simulink model "HvacCtrlrAirFlowDistbnFrnt"  (R2022b)
settings: Solver=FixedStepDiscrete  StartTime=0.0  StopTime=10.0  FixedStep=0.2  RelTol=1e-3
(part 1 of 2)
settings Model: Creator=blue.ge  LastModifiedBy=blue.ge
settings DataIOCC: SaveFormat=StructureWithTime
settings OptimizationCC: DefaultParameterBehavior=Inlined  EnhancedBackFolding=on  PassReuseOutputArgsAs=Individual arguments  ZeroExternalMemoryAtStartup=off  ZeroInternalMemoryAtStartup=off  OptimizeBlockOrder=speed  DifferentSizesBufferReuse=on  OptimizationCustomize=off  EfficientTunableParamExpr=on
settings DebuggingCC: ParameterTunabilityLossMsg=error
settings ModelReferenceCC: ModelReferenceNumInstancesAllowed=Single
settings SFSimCC: MATLABDynamicMemAlloc=off
settings RTWCC: Description=AUTOSAR  SystemTargetFile=autosar.tlc  GenCodeOnly=on  TemplateMakefile=ert_default_tmf
settings CodeAppCC: ForceParamTrailComments=on  IgnoreCustomStorageClasses=off  OperatorAnnotations=on  SimulinkDataObjDesc=on  SFDataObjDesc=on  InsertBlockDesc=on
settings STFCustomTargetCC: TargetLangStandard=C99 (ISO)  MultiwordLength=256  ParMdlRefBuildCompliant=on  CompOptLevelCompliant=on  IncludeMdlTerminateFcn=off  CombineOutputUpdateFcns=on  SuppressErrorStatus=on  ERTCustomFileBanners=on  SupportNonFinite=off  SupportComplex=off  SupportContinuousTime=off  SupportNonInlinedSFcns=off  RemoveResetFunc=on  MATLABClassNameForMDSCustomization=Simulink.SoftwareTarget.ERTCustomization  AutosarCompliant=on  UseToolchainInfoCompliant=on  LookupTableObjectStructAxisOrder=2,1,3,4,...  LUTObjectStructOrderEvenSpacing=Size,Table,Breakpoints  SystemTargetFile=autosar.tlc
settings ERTTargetCC: TargetLangStandard=C99 (ISO)  ConcurrentExecutionCompliant=off  IncludeMdlTerminateFcn=off  SuppressErrorStatus=on  SupportNonFinite=off  SupportComplex=off  ModelStepFunctionPrototypeControlCompliant=off  CPPClassGenCompliant=off  AutosarCompliant=on  LookupTableObjectStructAxisOrder=2,1,3,4,...  LUTObjectStructOrderEvenSpacing=Size,Table,Breakpoints  InlinedParameterPlacement=NonHierarchical  ERTCustomFileTemplate=example_file_process.tlc

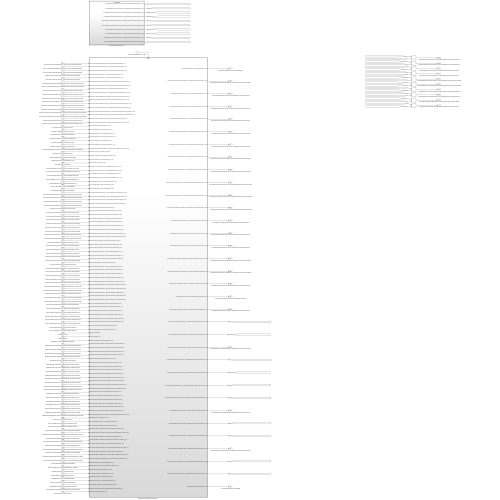
[thumbnail: Simulink's stored preview of the top-level diagram]
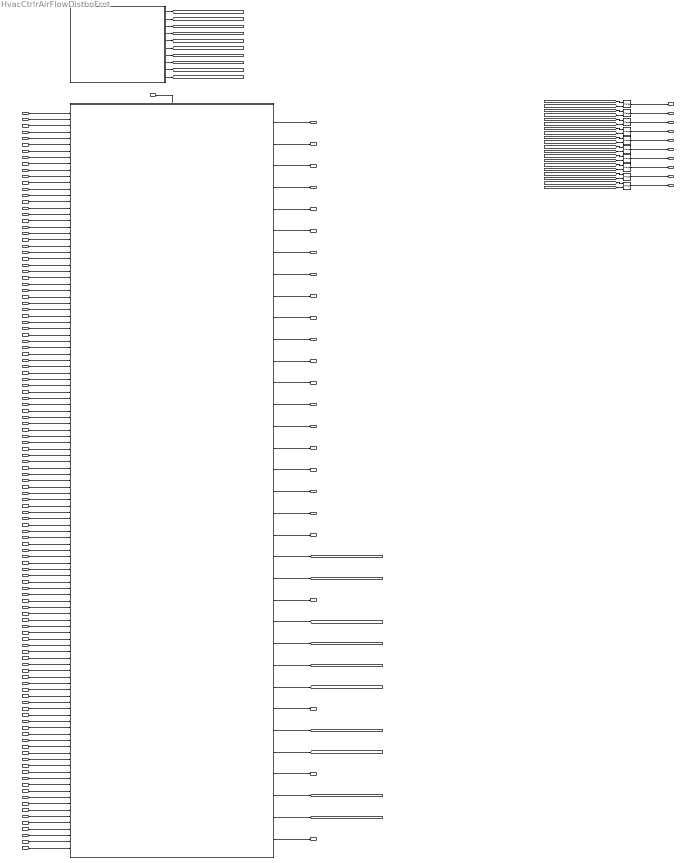
== system HvacCtrlrAirFlowDistbnFrnt ==
  HvacCtrlrAirFlowDistbnFrnt_Runnable : Inport  SampleTime=0.2  OutputFunctionCall=on
  ActrPosnFrntActForRowFirstModDistbn_ActrPosnFrntActForRowFirstModDistbn : Inport  Port=2  OutMin=0  OutMax=100  OutDataTypeStr=Perc9  PortDimensions=1
  ActrPosnFrntActForRowSecModDistbnLe_ActrPosnFrntActForRowSecModDistbnLe : Inport  Port=3  OutMin=0  OutMax=100  OutDataTypeStr=Perc9  PortDimensions=1
  ActrPosnFrntActForRowSecModDistbnRi_ActrPosnFrntActForRowSecModDistbnRi : Inport  Port=4  OutMin=0  OutMax=100  OutDataTypeStr=Perc9  PortDimensions=1
  ActrPosnMaxLimForVentForRowSec_ActrPosnMaxLimForVentForRowSec : Inport  Port=5  OutDataTypeStr=Perc30  PortDimensions=1
  ActrPosnMinLimForVentForRowSec_ActrPosnMinLimForVentForRowSec : Inport  Port=6  OutDataTypeStr=Perc30  PortDimensions=1
  ActrPosnReqForModDistbnForClimaCtrlMan_ActrPosnReqForModDistbnForClimaCtrlMan : Inport  Port=7  OutDataTypeStr=Perc30  PortDimensions=1
  AirFlapPosnFrntActForRowFirstForActrDefrst_AirFlapPosnFrntActForRowFirstForActrDefrst : Inport  Port=8  OutMin=0  OutMax=100  OutDataTypeStr=Perc9  PortDimensions=1
  AirFlapPosnFrntActForRowFirstForActrFlr_AirFlapPosnFrntActForRowFirstForActrFlr : Inport  Port=9  OutMin=0  OutMax=100  OutDataTypeStr=Perc9  PortDimensions=1
  AirFlapPosnFrntActForRowFirstForActrFlrLe_AirFlapPosnFrntActForRowFirstForActrFlrLe : Inport  Port=10  OutMin=0  OutMax=100  OutDataTypeStr=Perc9  PortDimensions=1
  AirFlapPosnFrntActForRowFirstForActrFlrRi_AirFlapPosnFrntActForRowFirstForActrFlrRi : Inport  Port=11  OutMin=0  OutMax=100  OutDataTypeStr=Perc9  PortDimensions=1
  AirFlapPosnFrntActForRowFirstForActrVent_AirFlapPosnFrntActForRowFirstForActrVent : Inport  Port=12  OutMin=0  OutMax=100  OutDataTypeStr=Perc9  PortDimensions=1
  AirFlapPosnFrntActForRowFirstForActrVentLe_AirFlapPosnFrntActForRowFirstForActrVentLe : Inport  Port=13  OutMin=0  OutMax=100  OutDataTypeStr=Perc9  PortDimensions=1
  AirFlapPosnFrntActForRowFirstForActrVentRi_AirFlapPosnFrntActForRowFirstForActrVentRi : Inport  Port=14  OutMin=0  OutMax=100  OutDataTypeStr=Perc9  PortDimensions=1
  AirFlapPosnFrntActForRowFirstForActrVentSideLe_AirFlapPosnFrntActForRowFirstForActrVentSideLe : Inport  Port=15  OutMin=0  OutMax=100  OutDataTypeStr=Perc9  PortDimensions=1
  AirFlapPosnFrntActForRowFirstForActrVentSideRi_AirFlapPosnFrntActForRowFirstForActrVentSideRi : Inport  Port=16  OutMin=0  OutMax=100  OutDataTypeStr=Perc9  PortDimensions=1
  AirFlapPosnFrntActForRowSecForActrFlr_AirFlapPosnFrntActForRowSecForActrFlr : Inport  Port=17  OutMin=0  OutMax=100  OutDataTypeStr=Perc9  PortDimensions=1
  AirFlapPosnFrntActForRowSecForActrVent_AirFlapPosnFrntActForRowSecForActrVent : Inport  Port=18  OutMin=0  OutMax=100  OutDataTypeStr=Perc9  PortDimensions=1
  AmbTFildForClimaNgcc_AmbTFildForClima : Inport  Port=19  OutDataTypeStr=T30  PortDimensions=1
  ClimaCfgForActrModVrnt1_ClimaCfgForActrInd1 : Inport  Port=20  OutDataTypeStr=Enum: ClimaCfgForActrInd1  PortDimensions=1
  ClimaCfgForActrModVrnt1_ClimaCfgForActrModVrnt1 : Inport  Port=21  OutDataTypeStr=Enum: ClimaCfgForActrModVrnt1  PortDimensions=1
  ClimaCfgForActrModVrnt1_ClimaCfgForActrModVrntCen : Inport  Port=22  OutDataTypeStr=Enum: ClimaCfgForActrModVrntCen  PortDimensions=1
  ClimaCfgForActrModVrnt2_ClimaCfgForActrInd2 : Inport  Port=23  OutDataTypeStr=Enum: ClimaCfgForActrInd2  PortDimensions=1
  ClimaCfgForActrModVrnt2_ClimaCfgForActrModVrnt2 : Inport  Port=24  OutDataTypeStr=Enum: ClimaCfgForActrModVrnt2  PortDimensions=1
  ClimaCfgForBlowrInRowSecVentSidePrsnt1_ClimaCfgForBlowrInRowSecVentSidePrsnt1 : Inport  Port=25  OutDataTypeStr=Boolean  PortDimensions=1
  ClimaCfgForCarTyp1_ClimaCfgForCarTyp1 : Inport  Port=26  OutDataTypeStr=Enum: ClimaCfgForCarTyp1  PortDimensions=1
  ClimaCfgForClimaCtrlMan1_ClimaCfgForClimaCtrlMan1 : Inport  Port=27  OutDataTypeStr=Boolean  PortDimensions=1
  ClimaCfgForNrOfTSetg1_ClimaCfgForNrOfTSetg1 : Inport  Port=28  OutDataTypeStr=Enum: ClimaCfgForNrOfTSetg1  PortDimensions=1
  ClimaCtrlActvNgcc_ClimaCtrlActv : Inport  Port=29  OutDataTypeStr=Boolean  PortDimensions=1
  HmiCmptmtAirDistbnFrntForClima_HmiCmptmtAirDistbnFrntForClima : Inport  Port=30  OutDataTypeStr=Enum: HmiCmptmtAirDistbnFrnt  PortDimensions=1
  HmiCmptmtAirDistbnFrntForClima_HmiCmptmtAirDistbnFrntRiForClima : Inport  Port=31  OutDataTypeStr=Enum: HmiCmptmtAirDistbnFrnt  PortDimensions=1
  HmiCmptmtAirDistbnFrntForClima_HmiCmptmtAirDistbnReForClima : Inport  Port=32  OutDataTypeStr=Enum: HmiCmptmtAirDistbnFrnt  PortDimensions=1
  HmiCmptmtAirDistbnFrntForClima_HmiCmptmtAirDistbnReRiForClima : Inport  Port=33  OutDataTypeStr=Enum: HmiCmptmtAirDistbnFrnt  PortDimensions=1
  HvacAirMFlowEstimdForCtrlr_HvacAirMFlowEstimdForCtrlr : Inport  Port=34  OutDataTypeStr=AirFlow30  PortDimensions=1
  HvacCtrlrFanBoostReqLe_HvacCtrlrFanBoostReqLe : Inport  Port=35  OutDataTypeStr=Perc30  PortDimensions=1
  HvacCtrlrFanBoostReqRi_HvacCtrlrFanBoostReqRi : Inport  Port=36  OutDataTypeStr=Perc30  PortDimensions=1
  HvacCtrlrReqFrntForRowFirstForActrTLe_HvacCtrlrReqFrntForRowFirstForActrTLe : Inport  Port=37  OutDataTypeStr=Perc30  PortDimensions=1
  HvacCtrlrReqFrntForRowFirstForActrTRi_HvacCtrlrReqFrntForRowFirstForActrTRi : Inport  Port=38  OutDataTypeStr=Perc30  PortDimensions=1
  HvacCtrlrReqFrntForRowSecForActrTLe_HvacCtrlrReqFrntForRowSecForActrTLe : Inport  Port=39  OutDataTypeStr=Perc30  PortDimensions=1
  HvacCtrlrReqFrntForRowSecForActrTRi_HvacCtrlrReqFrntForRowSecForActrTRi : Inport  Port=40  OutDataTypeStr=Perc30  PortDimensions=1
  HvacFlowFrntActForDefrst_HvacFlowFrntActForDefrst : Inport  Port=41  OutDataTypeStr=AirFlow30  PortDimensions=1
  HvacFlowFrntActForRowFirstAtFlr_HvacFlowFrntActForRowFirstAtFlr : Inport  Port=42  OutDataTypeStr=AirFlow30  PortDimensions=1
  HvacFlowFrntActForRowFirstAtFlr_HvacFlowFrntActForRowFirstAtFlrLe : Inport  Port=43  OutDataTypeStr=AirFlow30  PortDimensions=1
  HvacFlowFrntActForRowFirstAtFlr_HvacFlowFrntActForRowFirstAtFlrRi : Inport  Port=44  OutDataTypeStr=AirFlow30  PortDimensions=1
  HvacFlowFrntActForRowFirstAtVent_HvacFlowFrntActForRowFirstAtVent : Inport  Port=45  OutDataTypeStr=AirFlow30  PortDimensions=1
  HvacFlowFrntActForRowFirstAtVent_HvacFlowFrntActForRowFirstAtVentLe : Inport  Port=46  OutDataTypeStr=AirFlow30  PortDimensions=1
  HvacFlowFrntActForRowFirstAtVent_HvacFlowFrntActForRowFirstAtVentRi : Inport  Port=47  OutDataTypeStr=AirFlow30  PortDimensions=1
  HvacFlowFrntActForRowFirstAtVent_HvacFlowFrntActForRowFirstAtVentSideLe : Inport  Port=48  OutDataTypeStr=AirFlow30  PortDimensions=1
  HvacFlowFrntActForRowFirstAtVent_HvacFlowFrntActForRowFirstAtVentSideRi : Inport  Port=49  OutDataTypeStr=AirFlow30  PortDimensions=1
  HvacFlowFrntActForRowSecAtFlr_HvacFlowFrntActForRowSecAtFlr : Inport  Port=50  OutDataTypeStr=AirFlow30  PortDimensions=1
  HvacFlowFrntActForRowSecAtFlr_HvacFlowFrntActForRowSecAtFlrLe : Inport  Port=51  OutDataTypeStr=AirFlow30  PortDimensions=1
  HvacFlowFrntActForRowSecAtFlr_HvacFlowFrntActForRowSecAtFlrRi : Inport  Port=52  OutDataTypeStr=AirFlow30  PortDimensions=1
  HvacFlowFrntActForRowSecAtVent_HvacFlowFrntActForRowSecAtVent : Inport  Port=53  OutDataTypeStr=AirFlow30  PortDimensions=1
  HvacFlowFrntActForRowSecAtVent_HvacFlowFrntActForRowSecAtVentLe : Inport  Port=54  OutDataTypeStr=AirFlow30  PortDimensions=1
  HvacFlowFrntActForRowSecAtVent_HvacFlowFrntActForRowSecAtVentRi : Inport  Port=55  OutDataTypeStr=AirFlow30  PortDimensions=1
  HvacFlowFrntReqForDefrst_HvacFlowFrntReqForDefrst : Inport  Port=56  OutDataTypeStr=AirFlow30  PortDimensions=1
  HvacFlowFrntReqForRowFirstAtFlr_HvacFlowFrntReqForRowFirstAtFlr : Inport  Port=57  OutDataTypeStr=AirFlow30  PortDimensions=1
  HvacFlowFrntReqForRowFirstAtFlr_HvacFlowFrntReqForRowFirstAtFlrLe : Inport  Port=58  OutDataTypeStr=AirFlow30  PortDimensions=1
  HvacFlowFrntReqForRowFirstAtFlr_HvacFlowFrntReqForRowFirstAtFlrRi : Inport  Port=59  OutDataTypeStr=AirFlow30  PortDimensions=1
  HvacFlowFrntReqForRowFirstAtVent_HvacFlowFrntReqForRowFirstAtVent : Inport  Port=60  OutDataTypeStr=AirFlow30  PortDimensions=1
  HvacFlowFrntReqForRowFirstAtVent_HvacFlowFrntReqForRowFirstAtVentLe : Inport  Port=61  OutDataTypeStr=AirFlow30  PortDimensions=1
  HvacFlowFrntReqForRowFirstAtVent_HvacFlowFrntReqForRowFirstAtVentLeMid : Inport  Port=62  OutDataTypeStr=AirFlow30  PortDimensions=1
  HvacFlowFrntReqForRowFirstAtVent_HvacFlowFrntReqForRowFirstAtVentLeSide : Inport  Port=63  OutDataTypeStr=AirFlow30  PortDimensions=1
  HvacFlowFrntReqForRowFirstAtVent_HvacFlowFrntReqForRowFirstAtVentRi : Inport  Port=64  OutDataTypeStr=AirFlow30  PortDimensions=1
  HvacFlowFrntReqForRowFirstAtVent_HvacFlowFrntReqForRowFirstAtVentRiMid : Inport  Port=65  OutDataTypeStr=AirFlow30  PortDimensions=1
  HvacFlowFrntReqForRowFirstAtVent_HvacFlowFrntReqForRowFirstAtVentRiSide : Inport  Port=66  OutDataTypeStr=AirFlow30  PortDimensions=1
  HvacFlowFrntReqForRowSecAtFlr_HvacFlowFrntReqForRowSecAtFlr : Inport  Port=67  OutDataTypeStr=AirFlow30  PortDimensions=1
  HvacFlowFrntReqForRowSecAtFlr_HvacFlowFrntReqForRowSecAtFlrLe : Inport  Port=68  OutDataTypeStr=AirFlow30  PortDimensions=1
  HvacFlowFrntReqForRowSecAtFlr_HvacFlowFrntReqForRowSecAtFlrRi : Inport  Port=69  OutDataTypeStr=AirFlow30  PortDimensions=1
  HvacFlowFrntReqForRowSecAtVent_HvacFlowFrntReqForRowSecAtVent : Inport  Port=70  OutDataTypeStr=AirFlow30  PortDimensions=1
  HvacFlowFrntReqForRowSecAtVent_HvacFlowFrntReqForRowSecAtVentLe : Inport  Port=71  OutDataTypeStr=AirFlow30  PortDimensions=1
  HvacFlowFrntReqForRowSecAtVent_HvacFlowFrntReqForRowSecAtVentRi : Inport  Port=72  OutDataTypeStr=AirFlow30  PortDimensions=1
  HvacFlrLeakReqForRowFirst_HvacFlrLeakReqForRowFirst : Inport  Port=73  OutDataTypeStr=Boolean  PortDimensions=1
  HvacFlrLeakReqForRowSec_HvacFlrLeakReqForRowSec : Inport  Port=74  OutDataTypeStr=Boolean  PortDimensions=1
  LdAdVal_CurrAdVal : Inport  Port=75  OutDataTypeStr=Bus: LdAd  PortDimensions=1  BusOutputAsStruct=on
  LdAdVal_ValidAdVal : Inport  Port=76  OutDataTypeStr=Bus: LdAd  PortDimensions=1  BusOutputAsStruct=on
  ManModDurgFanOffActv_ManModDurgFanOffActv : Inport  Port=77  OutDataTypeStr=Boolean  PortDimensions=1
  ManModeForRowFirstLeForModelDial_ManModeForRowFirstLeForModelDial : Inport  Port=78  OutDataTypeStr=Enum: HmiCmptmtAirDistbnFrnt  PortDimensions=1
  ManModeForRowFirstRiForModelDial_ManModeForRowFirstRiForModelDial : Inport  Port=79  OutDataTypeStr=Enum: HmiCmptmtAirDistbnFrnt  PortDimensions=1
  ManModeForRowSecLeForModelDial_ManModeForRowSecLeForModelDial : Inport  Port=80  OutDataTypeStr=Enum: HmiCmptmtAirDistbnFrnt  PortDimensions=1
  ManModeForRowSecRiForModelDial_ManModeForRowSecRiForModelDial : Inport  Port=81  OutDataTypeStr=Enum: HmiCmptmtAirDistbnFrnt  PortDimensions=1
  MaxReqReqdFrntForDefrst_MaxReqReqdFrntForDefrst : Inport  Port=82  OutDataTypeStr=Boolean  PortDimensions=1
  MaxReqReqdFrntForRowFirstAtFlr_MaxReqReqdFrntForRowFirstAtFlr : Inport  Port=83  OutDataTypeStr=Boolean  PortDimensions=1
  MaxReqReqdFrntForRowFirstAtFlr_MaxReqReqdFrntForRowFirstAtFlrLe : Inport  Port=84  OutDataTypeStr=Boolean  PortDimensions=1
  MaxReqReqdFrntForRowFirstAtFlr_MaxReqReqdFrntForRowFirstAtFlrRi : Inport  Port=85  OutDataTypeStr=Boolean  PortDimensions=1
  MaxReqReqdFrntForRowFirstAtVent_MaxReqReqdFrntForRowFirstAtVent : Inport  Port=86  OutDataTypeStr=Boolean  PortDimensions=1
  MaxReqReqdFrntForRowFirstAtVent_MaxReqReqdFrntForRowFirstAtVentLe : Inport  Port=87  OutDataTypeStr=Boolean  PortDimensions=1
  MaxReqReqdFrntForRowFirstAtVent_MaxReqReqdFrntForRowFirstAtVentRi : Inport  Port=88  OutDataTypeStr=Boolean  PortDimensions=1
  MaxReqReqdFrntForRowFirstAtVent_MaxReqReqdFrntForRowFirstAtVentSideLe : Inport  Port=89  OutDataTypeStr=Boolean  PortDimensions=1
  MaxReqReqdFrntForRowFirstAtVent_MaxReqReqdFrntForRowFirstAtVentSideRi : Inport  Port=90  OutDataTypeStr=Boolean  PortDimensions=1
  MaxReqReqdFrntForRowSecAtFlr_MaxReqReqdFrntForRowSecAtFlr : Inport  Port=91  OutDataTypeStr=Boolean  PortDimensions=1
  MaxReqReqdFrntForRowSecAtFlr_MaxReqReqdFrntForRowSecAtFlrLe : Inport  Port=92  OutDataTypeStr=Boolean  PortDimensions=1
  MaxReqReqdFrntForRowSecAtFlr_MaxReqReqdFrntForRowSecAtFlrRi : Inport  Port=93  OutDataTypeStr=Boolean  PortDimensions=1
  MaxReqReqdFrntForRowSecAtVent_MaxReqReqdFrntForRowSecAtVent : Inport  Port=94  OutDataTypeStr=Boolean  PortDimensions=1
  MaxReqReqdFrntForRowSecAtVent_MaxReqReqdFrntForRowSecAtVentLe : Inport  Port=95  OutDataTypeStr=Boolean  PortDimensions=1
  MaxReqReqdFrntForRowSecAtVent_MaxReqReqdFrntForRowSecAtVentRi : Inport  Port=96  OutDataTypeStr=Boolean  PortDimensions=1
  NotAllwdForMaxReqReqdForRowSecAtVent_NotAllwdForMaxReqReqdForRowSecAtVent : Inport  Port=97  OutDataTypeStr=Boolean  PortDimensions=1
  VehSpdLgtForClima_VehSpdLgtForClima : Inport  Port=98  OutDataTypeStr=Bus: VehSpdLgtForClima  PortDimensions=1  BusOutputAsStruct=on
  VehSunLoadAtRowFirstLeFild_VehSunLoadAtRowFirstLeFild : Inport  Port=99  OutDataTypeStr=SunLoad30  PortDimensions=1
  VehSunLoadAtRowFirstRiFild_VehSunLoadAtRowFirstRiFild : Inport  Port=100  OutDataTypeStr=SunLoad30  PortDimensions=1
  ActrFrntReqForRowFirstLeModDistbn_ActrFrntReqForRowFirstLeModDistbn : Inport  Port=101  OutMin=0  OutMax=100  OutDataTypeStr=Perc9  PortDimensions=1
  ActrFrntReqForRowFirstLeModDistbn_ActrFrntReqForRowFirstLeModDistbn_IsUpdated : Inport  Port=102  OutDataTypeStr=boolean  PortDimensions=1
  ActrFrntReqForRowFirstModDistbn_ActrFrntReqForRowFirstModDistbn : Inport  Port=103  OutMin=0  OutMax=100  OutDataTypeStr=Perc9  PortDimensions=1
  ActrFrntReqForRowFirstModDistbn_ActrFrntReqForRowFirstModDistbn_IsUpdated : Inport  Port=104  OutDataTypeStr=boolean  PortDimensions=1
  ActrFrntReqForRowSecModDistbnLe_ActrFrntReqForRowSecModDistbnLe : Inport  Port=105  OutMin=0  OutMax=100  OutDataTypeStr=Perc9  PortDimensions=1
  ActrFrntReqForRowSecModDistbnLe_ActrFrntReqForRowSecModDistbnLe_IsUpdated : Inport  Port=106  OutDataTypeStr=boolean  PortDimensions=1
  ActrFrntReqForRowSecModDistbnRi_ActrFrntReqForRowSecModDistbnRi : Inport  Port=107  OutMin=0  OutMax=100  OutDataTypeStr=Perc9  PortDimensions=1
  ActrFrntReqForRowSecModDistbnRi_ActrFrntReqForRowSecModDistbnRi_IsUpdated : Inport  Port=108  OutDataTypeStr=boolean  PortDimensions=1
  ActrPosnFrntActForRowFirstModDistbnLe_ActrPosnFrntActForRowFirstModDistbnLe : Inport  Port=109  OutMin=0  OutMax=100  OutDataTypeStr=Perc9  PortDimensions=1
  ActrReqForModDistbnRe_ActrReqForModDistbnRe : Inport  Port=110  OutMin=0  OutMax=100  OutDataTypeStr=Perc9  PortDimensions=1
  ActrReqForModDistbnRe_ActrReqForModDistbnRe_IsUpdated : Inport  Port=111  OutDataTypeStr=boolean  PortDimensions=1
  ClimaAtRowSecActvNgcc_ClimaAtRowSecActv : Inport  Port=112  OutDataTypeStr=Boolean  PortDimensions=1
  ClimaAtRowSecActvNgcc_ClimaAtRowSecActvLe : Inport  Port=113  OutDataTypeStr=Boolean  PortDimensions=1
  ClimaAtRowSecActvNgcc_ClimaAtRowSecActvRi : Inport  Port=114  OutDataTypeStr=Boolean  PortDimensions=1
  ClimaCfgForHvacRePrsnt1_ClimaCfgForHvacRePrsnt1 : Inport  Port=115  OutDataTypeStr=Enum: CarCfgForHvacRe  PortDimensions=1
  ClimaCfgReqVersionReplace_ClimaCfgReqVersionReplace : Inport  Port=116  OutDataTypeStr=UInt8  PortDimensions=1
  ManModeForRowThrdForModelDial_ManModeForRowThrdForModelDial : Inport  Port=117  OutDataTypeStr=Enum: HmiCmptmtAirDistbnFrnt  PortDimensions=1
  PrkgClimaOnReq_PrkgClimaOnReq : Inport  Port=118  OutDataTypeStr=Boolean  PortDimensions=1
  HvacCtrlrAirFlowDistbnFrnt_Init : Subsystem
  HvacCtrlrAirFlowDistbnFrnt_Runnable_sys : Subsystem
  OvrdForMaxReqForActrForRowFirstAtFlr_OvrdForMaxReqForActrForRowFirstAtFlrLe_merge : Merge
  OvrdForMaxReqForActrForRowFirstAtFlr_OvrdForMaxReqForActrForRowFirstAtFlrRi_merge : Merge
  OvrdForMaxReqForActrForRowFirstAtVent_OvrdForMaxReqForActrForRowFirstAtVentLe_merge : Merge
  OvrdForMaxReqForActrForRowFirstAtVent_OvrdForMaxReqForActrForRowFirstAtVentRi_merge : Merge
  OvrdForMaxReqForActrForRowFirstAtVent_OvrdForMaxReqForActrForRowFirstAtVentSideLe_merge : Merge
  OvrdForMaxReqForActrForRowFirstAtVent_OvrdForMaxReqForActrForRowFirstAtVentSideRi_merge : Merge
  OvrdForMaxReqForActrForRowSecAtFlr_OvrdForMaxReqForActrForRowSecAtFlrLe_merge : Merge
  OvrdForMaxReqForActrForRowSecAtFlr_OvrdForMaxReqForActrForRowSecAtFlrRi_merge : Merge
  OvrdForMaxReqForActrForRowSecAtVent_OvrdForMaxReqForActrForRowSecAtVentLe_merge : Merge
  OvrdForMaxReqForActrForRowSecAtVent_OvrdForMaxReqForActrForRowSecAtVentRi_merge : Merge
  OvrdForMaxReqForActrForRowFirstAtFlr_OvrdForMaxReqForActrForRowFirstAtFlrLe : Outport  OutDataTypeStr=Boolean  PortDimensions=1
  OvrdForMaxReqForActrForRowFirstAtFlr_OvrdForMaxReqForActrForRowFirstAtFlrRi : Outport  Port=2  OutDataTypeStr=Boolean  PortDimensions=1
  OvrdForMaxReqForActrForRowFirstAtVent_OvrdForMaxReqForActrForRowFirstAtVentLe : Outport  Port=3  OutDataTypeStr=Boolean  PortDimensions=1
  OvrdForMaxReqForActrForRowFirstAtVent_OvrdForMaxReqForActrForRowFirstAtVentRi : Outport  Port=4  OutDataTypeStr=Boolean  PortDimensions=1
  OvrdForMaxReqForActrForRowFirstAtVent_OvrdForMaxReqForActrForRowFirstAtVentSideLe : Outport  Port=5  OutDataTypeStr=Boolean  PortDimensions=1
  OvrdForMaxReqForActrForRowFirstAtVent_OvrdForMaxReqForActrForRowFirstAtVentSideRi : Outport  Port=6  OutDataTypeStr=Boolean  PortDimensions=1
  OvrdForMaxReqForActrForRowSecAtFlr_OvrdForMaxReqForActrForRowSecAtFlrLe : Outport  Port=7  OutDataTypeStr=Boolean  PortDimensions=1
  OvrdForMaxReqForActrForRowSecAtFlr_OvrdForMaxReqForActrForRowSecAtFlrRi : Outport  Port=8  OutDataTypeStr=Boolean  PortDimensions=1
  OvrdForMaxReqForActrForRowSecAtVent_OvrdForMaxReqForActrForRowSecAtVentLe : Outport  Port=9  OutDataTypeStr=Boolean  PortDimensions=1
  OvrdForMaxReqForActrForRowSecAtVent_OvrdForMaxReqForActrForRowSecAtVentRi : Outport  Port=10  OutDataTypeStr=Boolean  PortDimensions=1
  HvacAirMFlowReqMaxAvl_HvacAirMFlowReqMaxAvl : Outport  Port=11  OutDataTypeStr=AirFlow30  PortDimensions=1
  HvacCtrlrReqFrntForRowFirstForActrDefrst_HvacCtrlrReqFrntForRowFirstForActrDefrst : Outport  Port=12  OutDataTypeStr=Perc30  PortDimensions=1
  HvacCtrlrReqFrntForRowFirstForActrFlr_HvacCtrlrReqFrntForRowFirstForActrFlr : Outport  Port=13  OutDataTypeStr=Perc30  PortDimensions=1
  HvacCtrlrReqFrntForRowFirstForActrFlr_HvacCtrlrReqFrntForRowFirstForActrFlrLe : Outport  Port=14  OutDataTypeStr=Perc30  PortDimensions=1
  HvacCtrlrReqFrntForRowFirstForActrFlr_HvacCtrlrReqFrntForRowFirstForActrFlrRi : Outport  Port=15  OutDataTypeStr=Perc30  PortDimensions=1
  HvacCtrlrReqFrntForRowFirstForActrMod_HvacCtrlrReqFrntForRowFirstForActrMod : Outport  Port=16  OutDataTypeStr=Perc30  PortDimensions=1
  HvacCtrlrReqFrntForRowFirstForActrVent_HvacCtrlrReqFrntForRowFirstForActrVent : Outport  Port=17  OutDataTypeStr=Perc30  PortDimensions=1
  HvacCtrlrReqFrntForRowFirstForActrVent_HvacCtrlrReqFrntForRowFirstForActrVentLe : Outport  Port=18  OutDataTypeStr=Perc30  PortDimensions=1
  HvacCtrlrReqFrntForRowFirstForActrVent_HvacCtrlrReqFrntForRowFirstForActrVentRi : Outport  Port=19  OutDataTypeStr=Perc30  PortDimensions=1
  HvacCtrlrReqFrntForRowFirstForActrVent_HvacCtrlrReqFrntForRowFirstForActrVentSideLe : Outport  Port=20  OutDataTypeStr=Perc30  PortDimensions=1
  HvacCtrlrReqFrntForRowFirstForActrVent_HvacCtrlrReqFrntForRowFirstForActrVentSideRi : Outport  Port=21  OutDataTypeStr=Perc30  PortDimensions=1
  HvacCtrlrReqFrntForRowFirstLeForActrMod_HvacCtrlrReqFrntForRowFirstLeForActrMod : Outport  Port=22  OutDataTypeStr=Perc30  PortDimensions=1
  HvacCtrlrReqFrntForRowSecForActrFlr_HvacCtrlrReqFrntForRowSecForActrFlr : Outport  Port=23  OutDataTypeStr=Perc30  PortDimensions=1
  HvacCtrlrReqFrntForRowSecForActrFlr_HvacCtrlrReqFrntForRowSecForActrFlrLe : Outport  Port=24  OutDataTypeStr=Perc30  PortDimensions=1
  HvacCtrlrReqFrntForRowSecForActrFlr_HvacCtrlrReqFrntForRowSecForActrFlrRi : Outport  Port=25  OutDataTypeStr=Perc30  PortDimensions=1
  HvacCtrlrReqFrntForRowSecForActrModLe_HvacCtrlrReqFrntForRowSecForActrModLe : Outport  Port=26  OutDataTypeStr=Perc30  PortDimensions=1
  HvacCtrlrReqFrntForRowSecForActrModRi_HvacCtrlrReqFrntForRowSecForActrModRi : Outport  Port=27  OutDataTypeStr=Perc30  PortDimensions=1
  HvacCtrlrReqFrntForRowSecForActrVent_HvacCtrlrReqFrntForRowSecForActrVent : Outport  Port=28  OutDataTypeStr=Perc30  PortDimensions=1
  OvrdForMaxReqForActrForDefrst_OvrdForMaxReqForActrForDefrst : Outport  Port=29  OutDataTypeStr=Boolean  PortDimensions=1
  OvrdForMaxReqForActrForRowFirstAtFlr_OvrdForMaxReqForActrForRowFirstAtFlr : Outport  Port=30  OutDataTypeStr=Boolean  PortDimensions=1
  OvrdForMaxReqForActrForRowFirstAtVent_OvrdForMaxReqForActrForRowFirstAtVent : Outport  Port=31  OutDataTypeStr=Boolean  PortDimensions=1
  OvrdForMaxReqForActrForRowSecAtFlr_OvrdForMaxReqForActrForRowSecAtFlr : Outport  Port=32  OutDataTypeStr=Boolean  PortDimensions=1
  OvrdForMaxReqForActrForRowSecAtVent_OvrdForMaxReqForActrForRowSecAtVent : Outport  Port=33  OutDataTypeStr=Boolean  PortDimensions=1
  ResrvdSigForECM4_ResrvdSigForECM4 : Outport  Port=34  OutDataTypeStr=BattSnsrChksTbl  PortDimensions=1
  wires:
  ActrPosnFrntActForRowFirstModDistbn_ActrPosnFrntActForRowFirstModDistbn/1 -> HvacCtrlrAirFlowDistbnFrnt_Runnable_sys/1(ActrPosnFrntActForRowFirstModDistbn_ActrPosnFrntActForRowFirstModDistbn_read)
  ActrPosnFrntActForRowSecModDistbnLe_ActrPosnFrntActForRowSecModDistbnLe/1 -> HvacCtrlrAirFlowDistbnFrnt_Runnable_sys/2(ActrPosnFrntActForRowSecModDistbnLe_ActrPosnFrntActForRowSecModDistbnLe_read)
  ActrPosnFrntActForRowSecModDistbnRi_ActrPosnFrntActForRowSecModDistbnRi/1 -> HvacCtrlrAirFlowDistbnFrnt_Runnable_sys/3(ActrPosnFrntActForRowSecModDistbnRi_ActrPosnFrntActForRowSecModDistbnRi_read)
  ActrPosnMaxLimForVentForRowSec_ActrPosnMaxLimForVentForRowSec/1 -> HvacCtrlrAirFlowDistbnFrnt_Runnable_sys/4(ActrPosnMaxLimForVentForRowSec_ActrPosnMaxLimForVentForRowSec_read)
  ActrPosnMinLimForVentForRowSec_ActrPosnMinLimForVentForRowSec/1 -> HvacCtrlrAirFlowDistbnFrnt_Runnable_sys/5(ActrPosnMinLimForVentForRowSec_ActrPosnMinLimForVentForRowSec_read)
  ActrPosnReqForModDistbnForClimaCtrlMan_ActrPosnReqForModDistbnForClimaCtrlMan/1 -> HvacCtrlrAirFlowDistbnFrnt_Runnable_sys/6(ActrPosnReqForModDistbnForClimaCtrlMan_ActrPosnReqForModDistbnForClimaCtrlMan_read)
  AirFlapPosnFrntActForRowFirstForActrDefrst_AirFlapPosnFrntActForRowFirstForActrDefrst/1 -> HvacCtrlrAirFlowDistbnFrnt_Runnable_sys/7(AirFlapPosnFrntActForRowFirstForActrDefrst_AirFlapPosnFrntActForRowFirstForActrDefrst_read)
  AirFlapPosnFrntActForRowFirstForActrFlr_AirFlapPosnFrntActForRowFirstForActrFlr/1 -> HvacCtrlrAirFlowDistbnFrnt_Runnable_sys/8(AirFlapPosnFrntActForRowFirstForActrFlr_AirFlapPosnFrntActForRowFirstForActrFlr_read)
  AirFlapPosnFrntActForRowFirstForActrFlrLe_AirFlapPosnFrntActForRowFirstForActrFlrLe/1 -> HvacCtrlrAirFlowDistbnFrnt_Runnable_sys/9(AirFlapPosnFrntActForRowFirstForActrFlrLe_AirFlapPosnFrntActForRowFirstForActrFlrLe_read)
  AirFlapPosnFrntActForRowFirstForActrFlrRi_AirFlapPosnFrntActForRowFirstForActrFlrRi/1 -> HvacCtrlrAirFlowDistbnFrnt_Runnable_sys/10(AirFlapPosnFrntActForRowFirstForActrFlrRi_AirFlapPosnFrntActForRowFirstForActrFlrRi_read)
  AirFlapPosnFrntActForRowFirstForActrVent_AirFlapPosnFrntActForRowFirstForActrVent/1 -> HvacCtrlrAirFlowDistbnFrnt_Runnable_sys/11(AirFlapPosnFrntActForRowFirstForActrVent_AirFlapPosnFrntActForRowFirstForActrVent_read)
  AirFlapPosnFrntActForRowFirstForActrVentLe_AirFlapPosnFrntActForRowFirstForActrVentLe/1 -> HvacCtrlrAirFlowDistbnFrnt_Runnable_sys/12(AirFlapPosnFrntActForRowFirstForActrVentLe_AirFlapPosnFrntActForRowFirstForActrVentLe_read)
  AirFlapPosnFrntActForRowFirstForActrVentRi_AirFlapPosnFrntActForRowFirstForActrVentRi/1 -> HvacCtrlrAirFlowDistbnFrnt_Runnable_sys/13(AirFlapPosnFrntActForRowFirstForActrVentRi_AirFlapPosnFrntActForRowFirstForActrVentRi_read)
  AirFlapPosnFrntActForRowFirstForActrVentSideLe_AirFlapPosnFrntActForRowFirstForActrVentSideLe/1 -> HvacCtrlrAirFlowDistbnFrnt_Runnable_sys/14(AirFlapPosnFrntActForRowFirstForActrVentSideLe_AirFlapPosnFrntActForRowFirstForActrVentSideLe_read)
  AirFlapPosnFrntActForRowFirstForActrVentSideRi_AirFlapPosnFrntActForRowFirstForActrVentSideRi/1 -> HvacCtrlrAirFlowDistbnFrnt_Runnable_sys/15(AirFlapPosnFrntActForRowFirstForActrVentSideRi_AirFlapPosnFrntActForRowFirstForActrVentSideRi_read)
  AirFlapPosnFrntActForRowSecForActrFlr_AirFlapPosnFrntActForRowSecForActrFlr/1 -> HvacCtrlrAirFlowDistbnFrnt_Runnable_sys/16(AirFlapPosnFrntActForRowSecForActrFlr_AirFlapPosnFrntActForRowSecForActrFlr_read)
  AirFlapPosnFrntActForRowSecForActrVent_AirFlapPosnFrntActForRowSecForActrVent/1 -> HvacCtrlrAirFlowDistbnFrnt_Runnable_sys/17(AirFlapPosnFrntActForRowSecForActrVent_AirFlapPosnFrntActForRowSecForActrVent_read)
  AmbTFildForClimaNgcc_AmbTFildForClima/1 -> HvacCtrlrAirFlowDistbnFrnt_Runnable_sys/18(AmbTFildForClimaNgcc_AmbTFildForClima_read)
  ClimaCfgForActrModVrnt1_ClimaCfgForActrInd1/1 -> HvacCtrlrAirFlowDistbnFrnt_Runnable_sys/19(ClimaCfgForActrModVrnt1_ClimaCfgForActrInd1_read)
  ClimaCfgForActrModVrnt1_ClimaCfgForActrModVrnt1/1 -> HvacCtrlrAirFlowDistbnFrnt_Runnable_sys/20(ClimaCfgForActrModVrnt1_ClimaCfgForActrModVrnt1_read)
  ClimaCfgForActrModVrnt1_ClimaCfgForActrModVrntCen/1 -> HvacCtrlrAirFlowDistbnFrnt_Runnable_sys/21(ClimaCfgForActrModVrnt1_ClimaCfgForActrModVrntCen_read)
  ClimaCfgForActrModVrnt2_ClimaCfgForActrInd2/1 -> HvacCtrlrAirFlowDistbnFrnt_Runnable_sys/22(ClimaCfgForActrModVrnt2_ClimaCfgForActrInd2_read)
  ClimaCfgForActrModVrnt2_ClimaCfgForActrModVrnt2/1 -> HvacCtrlrAirFlowDistbnFrnt_Runnable_sys/23(ClimaCfgForActrModVrnt2_ClimaCfgForActrModVrnt2_read)
  ClimaCfgForBlowrInRowSecVentSidePrsnt1_ClimaCfgForBlowrInRowSecVentSidePrsnt1/1 -> HvacCtrlrAirFlowDistbnFrnt_Runnable_sys/24(ClimaCfgForBlowrInRowSecVentSidePrsnt1_ClimaCfgForBlowrInRowSecVentSidePrsnt1_read)
  ClimaCfgForCarTyp1_ClimaCfgForCarTyp1/1 -> HvacCtrlrAirFlowDistbnFrnt_Runnable_sys/25(ClimaCfgForCarTyp1_ClimaCfgForCarTyp1_read)